simulink model "control"  (R2020b)
settings: Solver=VariableStepAuto  StartTime=0.0  StopTime=20  FixedStep=0.0001  RelTol=1e-3
PreLoadFcn:
  | load('data.mat');
model workspace (MAT data in the model file):
  LUT = [0.000510726493303 0.00051026523397 0.000510216027351 0.00051017496753 0.000510239529035 0.000510188586418 0.000510178918773 0.000510209816127 0.000510206521938 0.000510199701921 0.000510191190244 0.000510212888059 ... (5994000 elements, 999x6000)]
  V0step = 0.1
  apogee = 700
  force = 710
  k_max = 0.01
  kstep = 0.005
undefined symbols (not defined in the file or in its folder): m
libraries: pid_lib x1
(part 2 of 2)

== matlab function control/MATLAB Function ==
  data: k_max input; k_min input; k_comp output; h input; h_set input
function k_comp = fcn(k_max, k_min, h, h_set)
k_comp = (k_max - k_min)*exp(-1*((h_set - h)/h_set)) + k_min;
